simulink model "pfb_float_fft_1k_sim"  (R2016b) (continued)
== system pfb_float_fft_1k_sim == (continued)
      mask help:
      | An explicitly laid-out delay line to avoid mapping to SRL16s
      | for use in pipelining to help achieve timing closure.
      mask init:
      | pipeline_init(gcb,'latency',latency);
      settings: AttributesFormatString=Latency = %<latency>
      mask Display:
      | port_label('input', 1, 'd');
      | port_label('output', 1, 'q');
      | disp(['Z^-', num2str(latency)]);
  pipeline31 : Subsystem  AncestorBlock=casper_library_delays/pipeline  mask(pipeline){latency=2}
      mask help:
      | An explicitly laid-out delay line to avoid mapping to SRL16s
      | for use in pipelining to help achieve timing closure.
      mask init:
      | pipeline_init(gcb,'latency',latency);
      settings: AttributesFormatString=Latency = %<latency>
      mask Display:
      | port_label('input', 1, 'd');
      | port_label('output', 1, 'q');
      | disp(['Z^-', num2str(latency)]);
  pipeline34 : Subsystem  AncestorBlock=casper_library_delays/pipeline  mask(pipeline){latency=1}
      mask help:
      | An explicitly laid-out delay line to avoid mapping to SRL16s
      | for use in pipelining to help achieve timing closure.
      mask init:
      | pipeline_init(gcb,'latency',latency);
      settings: AttributesFormatString=Latency = %<latency>
      mask Display:
      | port_label('input', 1, 'd');
      | port_label('output', 1, 'q');
      | disp(['Z^-', num2str(latency)]);
  pipeline35 : Subsystem  AncestorBlock=casper_library_delays/pipeline  mask(pipeline){latency=1}
      mask help:
      | An explicitly laid-out delay line to avoid mapping to SRL16s
      | for use in pipelining to help achieve timing closure.
      mask init:
      | pipeline_init(gcb,'latency',latency);
      settings: AttributesFormatString=Latency = %<latency>
      mask Display:
      | port_label('input', 1, 'd');
      | port_label('output', 1, 'q');
      | disp(['Z^-', num2str(latency)]);
  pipeline36 : Subsystem  AncestorBlock=casper_library_delays/pipeline  mask(pipeline){latency=2}
      mask help:
      | An explicitly laid-out delay line to avoid mapping to SRL16s
      | for use in pipelining to help achieve timing closure.
      mask init:
      | pipeline_init(gcb,'latency',latency);
      settings: AttributesFormatString=Latency = %<latency>
      mask Display:
      | port_label('input', 1, 'd');
      | port_label('output', 1, 'q');
      | disp(['Z^-', num2str(latency)]);
  pipeline38 : Subsystem  AncestorBlock=casper_library_delays/pipeline  mask(pipeline){latency=2}
      mask help:
      | An explicitly laid-out delay line to avoid mapping to SRL16s
      | for use in pipelining to help achieve timing closure.
      mask init:
      | pipeline_init(gcb,'latency',latency);
      settings: AttributesFormatString=Latency = %<latency>
      mask Display:
      | port_label('input', 1, 'd');
      | port_label('output', 1, 'q');
      | disp(['Z^-', num2str(latency)]);
  pipeline39 : Subsystem  AncestorBlock=casper_library_delays/pipeline  mask(pipeline){latency=2}
      mask help:
      | An explicitly laid-out delay line to avoid mapping to SRL16s
      | for use in pipelining to help achieve timing closure.
      mask init:
      | pipeline_init(gcb,'latency',latency);
      settings: AttributesFormatString=Latency = %<latency>
      mask Display:
      | port_label('input', 1, 'd');
      | port_label('output', 1, 'q');
      | disp(['Z^-', num2str(latency)]);
  pipeline4 : Subsystem  AncestorBlock=casper_library_delays/pipeline  mask(pipeline){latency=250}
      mask help:
      | An explicitly laid-out delay line to avoid mapping to SRL16s
      | for use in pipelining to help achieve timing closure.
      mask init:
      | pipeline_init(gcb,'latency',latency);
      settings: AttributesFormatString=Latency = %<latency>
      mask Display:
      | port_label('input', 1, 'd');
      | port_label('output', 1, 'q');
      | disp(['Z^-', num2str(latency)]);
  pipeline40 : Subsystem  AncestorBlock=casper_library_delays/pipeline  mask(pipeline){latency=2}
      mask help:
      | An explicitly laid-out delay line to avoid mapping to SRL16s
      | for use in pipelining to help achieve timing closure.
      mask init:
      | pipeline_init(gcb,'latency',latency);
      settings: AttributesFormatString=Latency = %<latency>
      mask Display:
      | port_label('input', 1, 'd');
      | port_label('output', 1, 'q');
      | disp(['Z^-', num2str(latency)]);
  pipeline41 : Subsystem  AncestorBlock=casper_library_delays/pipeline  mask(pipeline){latency=2}
      mask help:
      | An explicitly laid-out delay line to avoid mapping to SRL16s
      | for use in pipelining to help achieve timing closure.
      mask init:
      | pipeline_init(gcb,'latency',latency);
      settings: AttributesFormatString=Latency = %<latency>
      mask Display:
      | port_label('input', 1, 'd');
      | port_label('output', 1, 'q');
      | disp(['Z^-', num2str(latency)]);
  pipeline42 : Subsystem  AncestorBlock=casper_library_delays/pipeline  mask(pipeline){latency=2}
      mask help:
      | An explicitly laid-out delay line to avoid mapping to SRL16s
      | for use in pipelining to help achieve timing closure.
      mask init:
      | pipeline_init(gcb,'latency',latency);
      settings: AttributesFormatString=Latency = %<latency>
      mask Display:
      | port_label('input', 1, 'd');
      | port_label('output', 1, 'q');
      | disp(['Z^-', num2str(latency)]);
  pipeline43 : Subsystem  AncestorBlock=casper_library_delays/pipeline  mask(pipeline){latency=2}
      mask help:
      | An explicitly laid-out delay line to avoid mapping to SRL16s
      | for use in pipelining to help achieve timing closure.
      mask init:
      | pipeline_init(gcb,'latency',latency);
      settings: AttributesFormatString=Latency = %<latency>
      mask Display:
      | port_label('input', 1, 'd');
      | port_label('output', 1, 'q');
      | disp(['Z^-', num2str(latency)]);
  pipeline44 : Subsystem  AncestorBlock=casper_library_delays/pipeline  mask(pipeline){latency=2}
      mask help:
      | An explicitly laid-out delay line to avoid mapping to SRL16s
      | for use in pipelining to help achieve timing closure.
      mask init:
      | pipeline_init(gcb,'latency',latency);
      settings: AttributesFormatString=Latency = %<latency>
      mask Display:
      | port_label('input', 1, 'd');
      | port_label('output', 1, 'q');
      | disp(['Z^-', num2str(latency)]);
  pipeline45 : Subsystem  AncestorBlock=casper_library_delays/pipeline  mask(pipeline){latency=2}
      mask help:
      | An explicitly laid-out delay line to avoid mapping to SRL16s
      | for use in pipelining to help achieve timing closure.
      mask init:
      | pipeline_init(gcb,'latency',latency);
      settings: AttributesFormatString=Latency = %<latency>
      mask Display:
      | port_label('input', 1, 'd');
      | port_label('output', 1, 'q');
      | disp(['Z^-', num2str(latency)]);
  pipeline46 : Subsystem  AncestorBlock=casper_library_delays/pipeline  mask(pipeline){latency=2}
      mask help:
      | An explicitly laid-out delay line to avoid mapping to SRL16s
      | for use in pipelining to help achieve timing closure.
      mask init:
      | pipeline_init(gcb,'latency',latency);
      settings: AttributesFormatString=Latency = %<latency>
      mask Display:
      | port_label('input', 1, 'd');
      | port_label('output', 1, 'q');
      | disp(['Z^-', num2str(latency)]);
  pipeline47 : Subsystem  AncestorBlock=casper_library_delays/pipeline  mask(pipeline){latency=2}
      mask help:
      | An explicitly laid-out delay line to avoid mapping to SRL16s
      | for use in pipelining to help achieve timing closure.
      mask init:
      | pipeline_init(gcb,'latency',latency);
      settings: AttributesFormatString=Latency = %<latency>
      mask Display:
      | port_label('input', 1, 'd');
      | port_label('output', 1, 'q');
      | disp(['Z^-', num2str(latency)]);
  pipeline48 : Subsystem  AncestorBlock=casper_library_delays/pipeline  mask(pipeline){latency=2}
      mask help:
      | An explicitly laid-out delay line to avoid mapping to SRL16s
      | for use in pipelining to help achieve timing closure.
      mask init:
      | pipeline_init(gcb,'latency',latency);
      settings: AttributesFormatString=Latency = %<latency>
      mask Display:
      | port_label('input', 1, 'd');
      | port_label('output', 1, 'q');
      | disp(['Z^-', num2str(latency)]);
  pipeline5 : Subsystem  AncestorBlock=casper_library_delays/pipeline  mask(pipeline){latency=2}
      mask help:
      | An explicitly laid-out delay line to avoid mapping to SRL16s
      | for use in pipelining to help achieve timing closure.
      mask init:
      | pipeline_init(gcb,'latency',latency);
      settings: AttributesFormatString=Latency = %<latency>
      mask Display:
      | port_label('input', 1, 'd');
      | port_label('output', 1, 'q');
      | disp(['Z^-', num2str(latency)]);
  pipeline53 : Subsystem  AncestorBlock=casper_library_delays/pipeline  mask(pipeline){latency=2}
      mask help:
      | An explicitly laid-out delay line to avoid mapping to SRL16s
      | for use in pipelining to help achieve timing closure.
      mask init:
      | pipeline_init(gcb,'latency',latency);
      settings: AttributesFormatString=Latency = %<latency>
      mask Display:
      | port_label('input', 1, 'd');
      | port_label('output', 1, 'q');
      | disp(['Z^-', num2str(latency)]);
  pipeline55 : Subsystem  AncestorBlock=casper_library_delays/pipeline  mask(pipeline){latency=2}
      mask help:
      | An explicitly laid-out delay line to avoid mapping to SRL16s
      | for use in pipelining to help achieve timing closure.
      mask init:
      | pipeline_init(gcb,'latency',latency);
      settings: AttributesFormatString=Latency = %<latency>
      mask Display:
      | port_label('input', 1, 'd');
      | port_label('output', 1, 'q');
      | disp(['Z^-', num2str(latency)]);
  pipeline56 : Subsystem  AncestorBlock=casper_library_delays/pipeline  mask(pipeline){latency=2}
      mask help:
      | An explicitly laid-out delay line to avoid mapping to SRL16s
      | for use in pipelining to help achieve timing closure.
      mask init:
      | pipeline_init(gcb,'latency',latency);
      settings: AttributesFormatString=Latency = %<latency>
      mask Display:
      | port_label('input', 1, 'd');
      | port_label('output', 1, 'q');
      | disp(['Z^-', num2str(latency)]);
  pipeline57 : Subsystem  AncestorBlock=casper_library_delays/pipeline  mask(pipeline){latency=1}
      mask help:
      | An explicitly laid-out delay line to avoid mapping to SRL16s
      | for use in pipelining to help achieve timing closure.
      mask init:
      | pipeline_init(gcb,'latency',latency);
      settings: AttributesFormatString=Latency = %<latency>
      mask Display:
      | port_label('input', 1, 'd');
      | port_label('output', 1, 'q');
      | disp(['Z^-', num2str(latency)]);
  pipeline58 : Subsystem  AncestorBlock=casper_library_delays/pipeline  mask(pipeline){latency=2}
      mask help:
      | An explicitly laid-out delay line to avoid mapping to SRL16s
      | for use in pipelining to help achieve timing closure.
      mask init:
      | pipeline_init(gcb,'latency',latency);
      settings: AttributesFormatString=Latency = %<latency>
      mask Display:
      | port_label('input', 1, 'd');
      | port_label('output', 1, 'q');
      | disp(['Z^-', num2str(latency)]);
  pipeline59 : Subsystem  AncestorBlock=casper_library_delays/pipeline  mask(pipeline){latency=2}
      mask help:
      | An explicitly laid-out delay line to avoid mapping to SRL16s
      | for use in pipelining to help achieve timing closure.
      mask init:
      | pipeline_init(gcb,'latency',latency);
      settings: AttributesFormatString=Latency = %<latency>
      mask Display:
      | port_label('input', 1, 'd');
      | port_label('output', 1, 'q');
      | disp(['Z^-', num2str(latency)]);
  pipeline6 : Subsystem  AncestorBlock=casper_library_delays/pipeline  mask(pipeline){latency=2}
      mask help:
      | An explicitly laid-out delay line to avoid mapping to SRL16s
      | for use in pipelining to help achieve timing closure.
      mask init:
      | pipeline_init(gcb,'latency',latency);
      settings: AttributesFormatString=Latency = %<latency>
      mask Display:
      | port_label('input', 1, 'd');
      | port_label('output', 1, 'q');
      | disp(['Z^-', num2str(latency)]);
  pipeline60 : Subsystem  AncestorBlock=casper_library_delays/pipeline  mask(pipeline){latency=2}
      mask help:
      | An explicitly laid-out delay line to avoid mapping to SRL16s
      | for use in pipelining to help achieve timing closure.
      mask init:
      | pipeline_init(gcb,'latency',latency);
      settings: AttributesFormatString=Latency = %<latency>
      mask Display:
      | port_label('input', 1, 'd');
      | port_label('output', 1, 'q');
      | disp(['Z^-', num2str(latency)]);
  pipeline61 : Subsystem  AncestorBlock=casper_library_delays/pipeline  mask(pipeline){latency=2}
      mask help:
      | An explicitly laid-out delay line to avoid mapping to SRL16s
      | for use in pipelining to help achieve timing closure.
      mask init:
      | pipeline_init(gcb,'latency',latency);
      settings: AttributesFormatString=Latency = %<latency>
      mask Display:
      | port_label('input', 1, 'd');
      | port_label('output', 1, 'q');
      | disp(['Z^-', num2str(latency)]);
  pipeline62 : Subsystem  AncestorBlock=casper_library_delays/pipeline  mask(pipeline){latency=1}
      mask help:
      | An explicitly laid-out delay line to avoid mapping to SRL16s
      | for use in pipelining to help achieve timing closure.
      mask init:
      | pipeline_init(gcb,'latency',latency);
      settings: AttributesFormatString=Latency = %<latency>
      mask Display:
      | port_label('input', 1, 'd');
      | port_label('output', 1, 'q');
      | disp(['Z^-', num2str(latency)]);
  pipeline63 : Subsystem  AncestorBlock=casper_library_delays/pipeline  mask(pipeline){latency=1}
      mask help:
      | An explicitly laid-out delay line to avoid mapping to SRL16s
      | for use in pipelining to help achieve timing closure.
      mask init:
      | pipeline_init(gcb,'latency',latency);
      settings: AttributesFormatString=Latency = %<latency>
      mask Display:
      | port_label('input', 1, 'd');
      | port_label('output', 1, 'q');
      | disp(['Z^-', num2str(latency)]);
  pipeline7 : Subsystem  AncestorBlock=casper_library_delays/pipeline  mask(pipeline){latency=2}
      mask help:
      | An explicitly laid-out delay line to avoid mapping to SRL16s
      | for use in pipelining to help achieve timing closure.
      mask init:
      | pipeline_init(gcb,'latency',latency);
      settings: AttributesFormatString=Latency = %<latency>
      mask Display:
      | port_label('input', 1, 'd');
      | port_label('output', 1, 'q');
      | disp(['Z^-', num2str(latency)]);
  pipeline79 : Subsystem  AncestorBlock=casper_library_delays/pipeline  mask(pipeline){latency=2}
      mask help:
      | An explicitly laid-out delay line to avoid mapping to SRL16s
      | for use in pipelining to help achieve timing closure.
      mask init:
      | pipeline_init(gcb,'latency',latency);
      settings: AttributesFormatString=Latency = %<latency>
      mask Display:
      | port_label('input', 1, 'd');
      | port_label('output', 1, 'q');
      | disp(['Z^-', num2str(latency)]);
  pipeline8 : Subsystem  AncestorBlock=casper_library_delays/pipeline  mask(pipeline){latency=2}
      mask help:
      | An explicitly laid-out delay line to avoid mapping to SRL16s
      | for use in pipelining to help achieve timing closure.
      mask init:
      | pipeline_init(gcb,'latency',latency);
      settings: AttributesFormatString=Latency = %<latency>
      mask Display:
      | port_label('input', 1, 'd');
      | port_label('output', 1, 'q');
      | disp(['Z^-', num2str(latency)]);
  pipeline80 : Subsystem  AncestorBlock=casper_library_delays/pipeline  mask(pipeline){latency=2}
      mask help:
      | An explicitly laid-out delay line to avoid mapping to SRL16s
      | for use in pipelining to help achieve timing closure.
      mask init:
      | pipeline_init(gcb,'latency',latency);
      settings: AttributesFormatString=Latency = %<latency>
      mask Display:
      | port_label('input', 1, 'd');
      | port_label('output', 1, 'q');
      | disp(['Z^-', num2str(latency)]);
  pipeline81 : Subsystem  AncestorBlock=casper_library_delays/pipeline  mask(pipeline){latency=2}
      mask help:
      | An explicitly laid-out delay line to avoid mapping to SRL16s
      | for use in pipelining to help achieve timing closure.
      mask init:
      | pipeline_init(gcb,'latency',latency);
      settings: AttributesFormatString=Latency = %<latency>
      mask Display:
      | port_label('input', 1, 'd');
      | port_label('output', 1, 'q');
      | disp(['Z^-', num2str(latency)]);
  pipeline82 : Subsystem  AncestorBlock=casper_library_delays/pipeline  mask(pipeline){latency=2}
      mask help:
      | An explicitly laid-out delay line to avoid mapping to SRL16s
      | for use in pipelining to help achieve timing closure.
      mask init:
      | pipeline_init(gcb,'latency',latency);
      settings: AttributesFormatString=Latency = %<latency>
      mask Display:
      | port_label('input', 1, 'd');
      | port_label('output', 1, 'q');
      | disp(['Z^-', num2str(latency)]);
  pipeline83 : Subsystem  AncestorBlock=casper_library_delays/pipeline  mask(pipeline){latency=2}
      mask help:
      | An explicitly laid-out delay line to avoid mapping to SRL16s
      | for use in pipelining to help achieve timing closure.
      mask init:
      | pipeline_init(gcb,'latency',latency);
      settings: AttributesFormatString=Latency = %<latency>
      mask Display:
      | port_label('input', 1, 'd');
      | port_label('output', 1, 'q');
      | disp(['Z^-', num2str(latency)]);
  pipeline84 : Subsystem  AncestorBlock=casper_library_delays/pipeline  mask(pipeline){latency=2}
      mask help:
      | An explicitly laid-out delay line to avoid mapping to SRL16s
      | for use in pipelining to help achieve timing closure.
      mask init:
      | pipeline_init(gcb,'latency',latency);
      settings: AttributesFormatString=Latency = %<latency>
      mask Display:
      | port_label('input', 1, 'd');
      | port_label('output', 1, 'q');
      | disp(['Z^-', num2str(latency)]);
  pipeline9 : Subsystem  AncestorBlock=casper_library_delays/pipeline  mask(pipeline){latency=2}
      mask help:
      | An explicitly laid-out delay line to avoid mapping to SRL16s
      | for use in pipelining to help achieve timing closure.
      mask init:
      | pipeline_init(gcb,'latency',latency);
      settings: AttributesFormatString=Latency = %<latency>
      mask Display:
      | port_label('input', 1, 'd');
      | port_label('output', 1, 'q');
      | disp(['Z^-', num2str(latency)]);
  pipeline96 : Subsystem  AncestorBlock=casper_library_delays/pipeline  mask(pipeline){latency=2}
      mask help:
      | An explicitly laid-out delay line to avoid mapping to SRL16s
      | for use in pipelining to help achieve timing closure.
      mask init:
      | pipeline_init(gcb,'latency',latency);
      settings: AttributesFormatString=Latency = %<latency>
      mask Display:
      | port_label('input', 1, 'd');
      | port_label('output', 1, 'q');
      | disp(['Z^-', num2str(latency)]);
  pipeline98 : Subsystem  AncestorBlock=casper_library_delays/pipeline  mask(pipeline){latency=2}
      mask help:
      | An explicitly laid-out delay line to avoid mapping to SRL16s
      | for use in pipelining to help achieve timing closure.
      mask init:
      | pipeline_init(gcb,'latency',latency);
      settings: AttributesFormatString=Latency = %<latency>
      mask Display:
      | port_label('input', 1, 'd');
      | port_label('output', 1, 'q');
      | disp(['Z^-', num2str(latency)]);
  pipeline99 : Subsystem  AncestorBlock=casper_library_delays/pipeline  mask(pipeline){latency=1}
      mask help:
      | An explicitly laid-out delay line to avoid mapping to SRL16s
      | for use in pipelining to help achieve timing closure.
      mask init:
      | pipeline_init(gcb,'latency',latency);
      settings: AttributesFormatString=Latency = %<latency>
      mask Display:
      | port_label('input', 1, 'd');
      | port_label('output', 1, 'q');
      | disp(['Z^-', num2str(latency)]);
  real_square{,1..3} (4 alike) : xbsIndex_r4/Mult  precision=Full  arith_type="Signed  (2's comp)"  n_bits=8  bin_pt=2  quantization=Truncate  overflow=Wrap  en=off  latency=6  use_behavioral_HDL=off  opt=Speed  use_embedded=on  optimum_pipeline=off  pipeline=on  dbl_ovrd=off  use_rpm=on  placement_style=Rectangular shape  block_type=mult
      settings: xl_area=[0, 0, 0, 0, 0, 0, 0]  block_version=9.1.01
  rpt_en_cwg0 : Subsystem  Tag=xps:sw_reg  AncestorBlock=xps_library/software_register  mask(swreg){io_dir=From Processor, io_delay=1, sample_period=1, names=[en dummy], bitwidths=[1 31], bin_pts=[0 0], arith_types=[0 0], sim_port=on, show_format=on}
      mask prompts: io_dir "I/O direction"; io_delay "I/O delay"; sample_period "Sample period"; names "Bitfield names [msb...lsb]"; bitwidths "Bitfield widths"; bin_pts "Bitfield binary pts"; arith_types "Bitfield types, ufix=0, fix=1, bool=2"; sim_port "Provide sim input/output?"; show_format "Print format string?"
      mask help: A 32-bit software-accessible register. Can be divided into bitfields of varying widths and types using the fields in the block mask.
      mask init:
      | swreg_init(gcb)
      settings: AttributesFormatString=2 inputs:uf1.0,uf31.0 = 32 bits
      mask TypeOptions io_dir: To Processor | From Processor
      mask Callback io_dir:
      | mask_enables = get_param(gcb, 'MaskEnables');
      | mask_names = get_param(gcb, 'MaskNames');
      | if strcmp(get_param(gcb, 'io_dir'), 'To Processor'),
      |     mask_enables{ismember(mask_names, 'sample_period')} = 'off';
      | else
      |     mask_enables{ismember(mask_names, 'sample_period')} = 'on';
      | end
      | set_param(gcb, 'MaskEnables', mask_enables);
  rpt_length_cwg0 : Subsystem  Tag=xps:sw_reg  AncestorBlock=xps_library/software_register  mask(swreg){io_dir=From Processor, io_delay=1, sample_period=1, names=cwg0_repeat_length, bitwidths=32, bin_pts=0, arith_types=0, sim_port=on, show_format=on}
      mask prompts: io_dir "I/O direction"; io_delay "I/O delay"; sample_period "Sample period"; names "Bitfield names [msb...lsb]"; bitwidths "Bitfield widths"; bin_pts "Bitfield binary pts"; arith_types "Bitfield types, ufix=0, fix=1, bool=2"; sim_port "Provide sim input/output?"; show_format "Print format string?"
      mask help: A 32-bit software-accessible register. Can be divided into bitfields of varying widths and types using the fields in the block mask.
      mask init:
      | swreg_init(gcb)
      settings: AttributesFormatString=1input:uf32.0 = 32 bits
      mask TypeOptions io_dir: To Processor | From Processor
      mask Callback io_dir:
      | mask_enables = get_param(gcb, 'MaskEnables');
      | mask_names = get_param(gcb, 'MaskNames');
      | if strcmp(get_param(gcb, 'io_dir'), 'To Processor'),
      |     mask_enables{ismember(mask_names, 'sample_period')} = 'off';
      | else
      |     mask_enables{ismember(mask_names, 'sample_period')} = 'on';
      | end
      | set_param(gcb, 'MaskEnables', mask_enables);
  scale_cwg0 : Subsystem  Tag=xps:sw_reg  AncestorBlock=xps_library/software_register  mask(swreg){io_dir=From Processor, io_delay=1, sample_period=1, names=[scale dummy], bitwidths=16, bin_pts=15, arith_types=0, sim_port=on, show_format=on}
      mask prompts: io_dir "I/O direction"; io_delay "I/O delay"; sample_period "Sample period"; names "Bitfield names [msb...lsb]"; bitwidths "Bitfield widths"; bin_pts "Bitfield binary pts"; arith_types "Bitfield types, ufix=0, fix=1, bool=2"; sim_port "Provide sim input/output?"; show_format "Print format string?"
      mask help: A 32-bit software-accessible register. Can be divided into bitfields of varying widths and types using the fields in the block mask.
      mask init:
      | swreg_init(gcb)
      settings: AttributesFormatString=2 inputs:uf16.15,uf16.15 = 32 bits
      mask TypeOptions io_dir: To Processor | From Processor
      mask Callback io_dir:
      | mask_enables = get_param(gcb, 'MaskEnables');
      | mask_names = get_param(gcb, 'MaskNames');
      | if strcmp(get_param(gcb, 'io_dir'), 'To Processor'),
      |     mask_enables{ismember(mask_names, 'sample_period')} = 'off';
      | else
      |     mask_enables{ismember(mask_names, 'sample_period')} = 'on';
      | end
      | set_param(gcb, 'MaskEnables', mask_enables);
  scale_float : Subsystem
  scale_float1 : Subsystem
  scale_float2 : Subsystem
  scale_float3 : Subsystem
  scale_wng0 : Subsystem  Tag=xps:sw_reg  AncestorBlock=xps_library/software_register  mask(swreg){io_dir=From Processor, io_delay=1, sample_period=1, names=[scale dummy], bitwidths=16, bin_pts=15, arith_types=0, sim_port=on, show_format=on}
      mask prompts: io_dir "I/O direction"; io_delay "I/O delay"; sample_period "Sample period"; names "Bitfield names [msb...lsb]"; bitwidths "Bitfield widths"; bin_pts "Bitfield binary pts"; arith_types "Bitfield types, ufix=0, fix=1, bool=2"; sim_port "Provide sim input/output?"; show_format "Print format string?"
      mask help: A 32-bit software-accessible register. Can be divided into bitfields of varying widths and types using the fields in the block mask.
      mask init:
      | swreg_init(gcb)
      settings: AttributesFormatString=2 inputs:uf16.15,uf16.15 = 32 bits
      mask TypeOptions io_dir: To Processor | From Processor
      mask Callback io_dir:
      | mask_enables = get_param(gcb, 'MaskEnables');
      | mask_names = get_param(gcb, 'MaskNames');
      | if strcmp(get_param(gcb, 'io_dir'), 'To Processor'),
      |     mask_enables{ismember(mask_names, 'sample_period')} = 'off';
      | else
      |     mask_enables{ismember(mask_names, 'sample_period')} = 'on';
      | end
      | set_param(gcb, 'MaskEnables', mask_enables);
  simple_bram_vacc : Subsystem  AncestorBlock=casper_library_accumulators/simple_bram_vacc  mask(simple_bram_vacc){vec_len=2^8, arith_type=Signed, n_bits=128, bin_pt=68}
      mask prompts: vec_len "Vector length:"; arith_type "Output type:"; n_bits "Bit width (output):"; bin_pt "Binary point (output):"
      mask help: This simple vector accumulator is based on a shift register. It outputs its previous accumulated vector after a new_acc pulse is received.
      mask init:
      | simple_bram_vacc_init(gcb, ...
      |     'vec_len', vec_len, ...
      |     'arith_type', arith_type, ...
      |     'n_bits', n_bits, ...
      |     'bin_pt', bin_pt);
      mask TypeOptions arith_type: Signed | Unsigned
  simple_bram_vacc1 : Subsystem  AncestorBlock=casper_library_accumulators/simple_bram_vacc  mask(simple_bram_vacc){vec_len=2^8, arith_type=Signed, n_bits=128, bin_pt=68}
      mask prompts: vec_len "Vector length:"; arith_type "Output type:"; n_bits "Bit width (output):"; bin_pt "Binary point (output):"
      mask help: This simple vector accumulator is based on a shift register. It outputs its previous accumulated vector after a new_acc pulse is received.
      mask init:
      | simple_bram_vacc_init(gcb, ...
      |     'vec_len', vec_len, ...
      |     'arith_type', arith_type, ...
      |     'n_bits', n_bits, ...
      |     'bin_pt', bin_pt);
      mask TypeOptions arith_type: Signed | Unsigned
  simple_bram_vacc2 : Subsystem  AncestorBlock=casper_library_accumulators/simple_bram_vacc  mask(simple_bram_vacc){vec_len=2^8, arith_type=Signed, n_bits=128, bin_pt=68}
      mask prompts: vec_len "Vector length:"; arith_type "Output type:"; n_bits "Bit width (output):"; bin_pt "Binary point (output):"
      mask help: This simple vector accumulator is based on a shift register. It outputs its previous accumulated vector after a new_acc pulse is received.
      mask init:
      | simple_bram_vacc_init(gcb, ...
      |     'vec_len', vec_len, ...
      |     'arith_type', arith_type, ...
      |     'n_bits', n_bits, ...
      |     'bin_pt', bin_pt);
      mask TypeOptions arith_type: Signed | Unsigned
  simple_bram_vacc3 : Subsystem  AncestorBlock=casper_library_accumulators/simple_bram_vacc  mask(simple_bram_vacc){vec_len=2^8, arith_type=Signed, n_bits=128, bin_pt=68}
      mask prompts: vec_len "Vector length:"; arith_type "Output type:"; n_bits "Bit width (output):"; bin_pt "Binary point (output):"
      mask help: This simple vector accumulator is based on a shift register. It outputs its previous accumulated vector after a new_acc pulse is received.
      mask init:
      | simple_bram_vacc_init(gcb, ...
      |     'vec_len', vec_len, ...
      |     'arith_type', arith_type, ...
      |     'n_bits', n_bits, ...
      |     'bin_pt', bin_pt);
      mask TypeOptions arith_type: Signed | Unsigned
  spect_cnt : xbsIndex_r4/Counter  cnt_type=Free Running  cnt_to=Inf  operation=Up  start_count=0  cnt_by_val=1  arith_type=Unsigned  n_bits=32  bin_pt=0  load_pin=off  rst=on  en=on  explicit_period=on  period=1  use_behavioral_HDL=off  implementation=DSP48  use_rpm=off  dbl_ovrd=off  block_type=counter
  spectrum_counter : Subsystem  Tag=xps:sw_reg  AncestorBlock=xps_library/software_register  mask(swreg){io_dir=To Processor, io_delay=0, sample_period=1, names=reg, bitwidths=32, bin_pts=0, arith_types=0, sim_port=on, show_format=on}
      mask prompts: io_dir "I/O direction"; io_delay "I/O delay"; sample_period "Sample period"; names "Bitfield names [msb...lsb]"; bitwidths "Bitfield widths"; bin_pts "Bitfield binary pts"; arith_types "Bitfield types, ufix=0, fix=1, bool=2"; sim_port "Provide sim input/output?"; show_format "Print format string?"
      mask help: A 32-bit software-accessible register. Can be divided into bitfields of varying widths and types using the fields in the block mask.
      mask init:
      | swreg_init(gcb)
      settings: AttributesFormatString=1output:uf32.0 = 32 bits
      mask TypeOptions io_dir: To Processor | From Processor
      mask Callback io_dir:
      | mask_enables = get_param(gcb, 'MaskEnables');
      | mask_names = get_param(gcb, 'MaskNames');
      | if strcmp(get_param(gcb, 'io_dir'), 'To Processor'),
      |     mask_enables{ismember(mask_names, 'sample_period')} = 'off';
      | else
      |     mask_enables{ismember(mask_names, 'sample_period')} = 'on';
      | end
      | set_param(gcb, 'MaskEnables', mask_enables);
  spectrum_limit : Subsystem  Tag=xps:sw_reg  AncestorBlock=xps_library/software_register  mask(swreg){io_dir=From Processor, io_delay=0, sample_period=1, names=limit, bitwidths=32, bin_pts=0, arith_types=0, sim_port=on, show_format=on}
      mask prompts: io_dir "I/O direction"; io_delay "I/O delay"; sample_period "Sample period"; names "Bitfield names [msb...lsb]"; bitwidths "Bitfield widths"; bin_pts "Bitfield binary pts"; arith_types "Bitfield types, ufix=0, fix=1, bool=2"; sim_port "Provide sim input/output?"; show_format "Print format string?"
      mask help: A 32-bit software-accessible register. Can be divided into bitfields of varying widths and types using the fields in the block mask.
      mask init:
      | swreg_init(gcb)
      settings: AttributesFormatString=1input:uf32.0 = 32 bits
      mask TypeOptions io_dir: To Processor | From Processor
      mask Callback io_dir:
      | mask_enables = get_param(gcb, 'MaskEnables');
      | mask_names = get_param(gcb, 'MaskNames');
      | if strcmp(get_param(gcb, 'io_dir'), 'To Processor'),
      |     mask_enables{ismember(mask_names, 'sample_period')} = 'off';
      | else
      |     mask_enables{ismember(mask_names, 'sample_period')} = 'on';
      | end
      | set_param(gcb, 'MaskEnables', mask_enables);
  ss_pfb_sq0 : Subsystem  Tag=casper:bitsnap  AncestorBlock=casper_library_scopes/bitfield_snapshot  mask(bitsnap){io_names=[pfb2_0], io_widths=[128], io_bps=[68], io_types=[1], show_format=on, snap_storage=bram, snap_dram_dimm=2, snap_dram_clock=250, snap_nsamples=8, snap_data_width=128, snap_offset=off, snap_circap=off, snap_value=off, snap_ext_arm=on, snap_provide_outputs=on, snap_use_dsp48=on, snap_delay=2, extra_names=[c d], extra_widths=[30 2], extra_bps=[0 0], extra_types=[0 0]}
      mask prompts: io_names "Names [msb...lsb]"; io_widths "Bitwidths [msb...lsb]"; io_bps "Binary pts [msb...lsb]"; io_types "Data types [msb...lsb], ufix=0, fix=1, bool=2"; show_format "Print format string?"; snap_storage "Storage medium"; snap_dram_dimm "DRAM dimm"; snap_dram_clock "DRAM clock rate"; snap_nsamples "Number of Samples (2^?)"; snap_data_width "Data width"; snap_offset "Start delay support"; snap_circap "Circular capture support"; snap_value "Extra value capture support"; snap_ext_arm "External arm support"; snap_provide_outputs "Provide outputs"; snap_use_dsp48 "Use DSP48s to implement counters"; snap_delay "Pre-snapshot delay"; extra_names "Names [msb...lsb]"; extra_widths "Widths [msb...lsb]"; extra_bps "Binary pts [msb...lsb]"; extra_types "Data types [msb...lsb], ufix=0, fix=1, bool=2"
      mask help:
      | A wrapped snapshot block. Allows specification of inputs to a snapblock word so the toolflow can automatically set up the scope.
      | See documentation for snapshot for explanation of parameters.
      mask init:
      | bitsnap_init(gcb);
      settings: AttributesFormatString=|
      | snap(1):f128.68 = 128 bits\n128 wide, 256 deep
      | debugID: 37190085
      mask TypeOptions snap_storage: bram | dram
      mask Callback snap_storage: bitsnap_callback();
      mask TypeOptions snap_dram_dimm: 1 | 2 | 3 | 4
      mask TypeOptions snap_dram_clock: 200 | 250 | 266 | 300 | 333
      mask TypeOptions snap_data_width: 8 | 16 | 32 | 64 | 128
      mask Callback snap_value: bitsnap_callback();
      mask Callback snap_ext_arm: bitsnap_callback();
      mask Callback snap_provide_outputs: bitsnap_callback();
  ss_pfb_sq1 : Subsystem  Tag=casper:bitsnap  AncestorBlock=casper_library_scopes/bitfield_snapshot  mask(bitsnap){io_names=[pfb2_0], io_widths=[128], io_bps=[68], io_types=[1], show_format=on, snap_storage=bram, snap_dram_dimm=2, snap_dram_clock=250, snap_nsamples=8, snap_data_width=128, snap_offset=off, snap_circap=off, snap_value=off, snap_ext_arm=off, snap_provide_outputs=on, snap_use_dsp48=on, snap_delay=2, extra_names=[c d], extra_widths=[30 2], extra_bps=[0 0], extra_types=[0 0]}
      mask prompts: io_names "Names [msb...lsb]"; io_widths "Bitwidths [msb...lsb]"; io_bps "Binary pts [msb...lsb]"; io_types "Data types [msb...lsb], ufix=0, fix=1, bool=2"; show_format "Print format string?"; snap_storage "Storage medium"; snap_dram_dimm "DRAM dimm"; snap_dram_clock "DRAM clock rate"; snap_nsamples "Number of Samples (2^?)"; snap_data_width "Data width"; snap_offset "Start delay support"; snap_circap "Circular capture support"; snap_value "Extra value capture support"; snap_ext_arm "External arm support"; snap_provide_outputs "Provide outputs"; snap_use_dsp48 "Use DSP48s to implement counters"; snap_delay "Pre-snapshot delay"; extra_names "Names [msb...lsb]"; extra_widths "Widths [msb...lsb]"; extra_bps "Binary pts [msb...lsb]"; extra_types "Data types [msb...lsb], ufix=0, fix=1, bool=2"
      mask help:
      | A wrapped snapshot block. Allows specification of inputs to a snapblock word so the toolflow can automatically set up the scope.
      | See documentation for snapshot for explanation of parameters.
      mask init:
      | bitsnap_init(gcb);
      settings: AttributesFormatString=|
      | snap(1):f128.68 = 128 bits\n128 wide, 256 deep
      | debugID: 37219876
      mask TypeOptions snap_storage: bram | dram
      mask Callback snap_storage: bitsnap_callback();
      mask TypeOptions snap_dram_dimm: 1 | 2 | 3 | 4
      mask TypeOptions snap_dram_clock: 200 | 250 | 266 | 300 | 333
      mask TypeOptions snap_data_width: 8 | 16 | 32 | 64 | 128
      mask Callback snap_value: bitsnap_callback();
      mask Callback snap_ext_arm: bitsnap_callback();
      mask Callback snap_provide_outputs: bitsnap_callback();
  ss_pfb_sq2 : Subsystem  Tag=casper:bitsnap  AncestorBlock=casper_library_scopes/bitfield_snapshot  mask(bitsnap){io_names=[pfb2_0], io_widths=[128], io_bps=[68], io_types=[1], show_format=on, snap_storage=bram, snap_dram_dimm=2, snap_dram_clock=250, snap_nsamples=8, snap_data_width=128, snap_offset=off, snap_circap=off, snap_value=off, snap_ext_arm=off, snap_provide_outputs=on, snap_use_dsp48=on, snap_delay=2, extra_names=[c d], extra_widths=[30 2], extra_bps=[0 0], extra_types=[0 0]}
      mask prompts: io_names "Names [msb...lsb]"; io_widths "Bitwidths [msb...lsb]"; io_bps "Binary pts [msb...lsb]"; io_types "Data types [msb...lsb], ufix=0, fix=1, bool=2"; show_format "Print format string?"; snap_storage "Storage medium"; snap_dram_dimm "DRAM dimm"; snap_dram_clock "DRAM clock rate"; snap_nsamples "Number of Samples (2^?)"; snap_data_width "Data width"; snap_offset "Start delay support"; snap_circap "Circular capture support"; snap_value "Extra value capture support"; snap_ext_arm "External arm support"; snap_provide_outputs "Provide outputs"; snap_use_dsp48 "Use DSP48s to implement counters"; snap_delay "Pre-snapshot delay"; extra_names "Names [msb...lsb]"; extra_widths "Widths [msb...lsb]"; extra_bps "Binary pts [msb...lsb]"; extra_types "Data types [msb...lsb], ufix=0, fix=1, bool=2"
      mask help:
      | A wrapped snapshot block. Allows specification of inputs to a snapblock word so the toolflow can automatically set up the scope.
      | See documentation for snapshot for explanation of parameters.
      mask init:
      | bitsnap_init(gcb);
      settings: AttributesFormatString=|
      | snap(1):f128.68 = 128 bits\n128 wide, 256 deep
      | debugID: 37249667
      mask TypeOptions snap_storage: bram | dram
      mask Callback snap_storage: bitsnap_callback();
      mask TypeOptions snap_dram_dimm: 1 | 2 | 3 | 4
      mask TypeOptions snap_dram_clock: 200 | 250 | 266 | 300 | 333
      mask TypeOptions snap_data_width: 8 | 16 | 32 | 64 | 128
      mask Callback snap_value: bitsnap_callback();
      mask Callback snap_ext_arm: bitsnap_callback();
      mask Callback snap_provide_outputs: bitsnap_callback();
  ss_pfb_sq3 : Subsystem  Tag=casper:bitsnap  AncestorBlock=casper_library_scopes/bitfield_snapshot  mask(bitsnap){io_names=[pfb2_0], io_widths=[128], io_bps=[68], io_types=[1], show_format=on, snap_storage=bram, snap_dram_dimm=2, snap_dram_clock=250, snap_nsamples=8, snap_data_width=128, snap_offset=off, snap_circap=off, snap_value=off, snap_ext_arm=off, snap_provide_outputs=on, snap_use_dsp48=on, snap_delay=2, extra_names=[c d], extra_widths=[30 2], extra_bps=[0 0], extra_types=[0 0]}
      mask prompts: io_names "Names [msb...lsb]"; io_widths "Bitwidths [msb...lsb]"; io_bps "Binary pts [msb...lsb]"; io_types "Data types [msb...lsb], ufix=0, fix=1, bool=2"; show_format "Print format string?"; snap_storage "Storage medium"; snap_dram_dimm "DRAM dimm"; snap_dram_clock "DRAM clock rate"; snap_nsamples "Number of Samples (2^?)"; snap_data_width "Data width"; snap_offset "Start delay support"; snap_circap "Circular capture support"; snap_value "Extra value capture support"; snap_ext_arm "External arm support"; snap_provide_outputs "Provide outputs"; snap_use_dsp48 "Use DSP48s to implement counters"; snap_delay "Pre-snapshot delay"; extra_names "Names [msb...lsb]"; extra_widths "Widths [msb...lsb]"; extra_bps "Binary pts [msb...lsb]"; extra_types "Data types [msb...lsb], ufix=0, fix=1, bool=2"
      mask help:
      | A wrapped snapshot block. Allows specification of inputs to a snapblock word so the toolflow can automatically set up the scope.
      | See documentation for snapshot for explanation of parameters.
      mask init:
      | bitsnap_init(gcb);
      settings: AttributesFormatString=|
      | snap(1):f128.68 = 128 bits\n128 wide, 256 deep
      | debugID: 37279458
      mask TypeOptions snap_storage: bram | dram
      mask Callback snap_storage: bitsnap_callback();
      mask TypeOptions snap_dram_dimm: 1 | 2 | 3 | 4
      mask TypeOptions snap_dram_clock: 200 | 250 | 266 | 300 | 333
      mask TypeOptions snap_data_width: 8 | 16 | 32 | 64 | 128
      mask Callback snap_value: bitsnap_callback();
      mask Callback snap_ext_arm: bitsnap_callback();
      mask Callback snap_provide_outputs: bitsnap_callback();
  sys_en : Subsystem  Tag=xps:sw_reg  AncestorBlock=xps_library/software_register  mask(swreg){io_dir=From Processor, io_delay=0, sample_period=1, names=en, bitwidths=1, bin_pts=0, arith_types=2, sim_port=on, show_format=on}
      mask prompts: io_dir "I/O direction"; io_delay "I/O delay"; sample_period "Sample period"; names "Bitfield names [msb...lsb]"; bitwidths "Bitfield widths"; bin_pts "Bitfield binary pts"; arith_types "Bitfield types, ufix=0, fix=1, bool=2"; sim_port "Provide sim input/output?"; show_format "Print format string?"
      mask help: A 32-bit software-accessible register. Can be divided into bitfields of varying widths and types using the fields in the block mask.
      mask init:
      | swreg_init(gcb)
      settings: AttributesFormatString=1input:b = 1 bits
      mask TypeOptions io_dir: To Processor | From Processor
      mask Callback io_dir:
      | mask_enables = get_param(gcb, 'MaskEnables');
      | mask_names = get_param(gcb, 'MaskNames');
      | if strcmp(get_param(gcb, 'io_dir'), 'To Processor'),
      |     mask_enables{ismember(mask_names, 'sample_period')} = 'off';
      | else
      |     mask_enables{ismember(mask_names, 'sample_period')} = 'on';
      | end
      | set_param(gcb, 'MaskEnables', mask_enables);
  sys_rst : Subsystem  Tag=xps:sw_reg  AncestorBlock=xps_library/software_register  mask(swreg){io_dir=From Processor, io_delay=0, sample_period=1, names=rst, bitwidths=1, bin_pts=0, arith_types=2, sim_port=on, show_format=on}
      mask prompts: io_dir "I/O direction"; io_delay "I/O delay"; sample_period "Sample period"; names "Bitfield names [msb...lsb]"; bitwidths "Bitfield widths"; bin_pts "Bitfield binary pts"; arith_types "Bitfield types, ufix=0, fix=1, bool=2"; sim_port "Provide sim input/output?"; show_format "Print format string?"
      mask help: A 32-bit software-accessible register. Can be divided into bitfields of varying widths and types using the fields in the block mask.
      mask init:
      | swreg_init(gcb)
      settings: AttributesFormatString=1input:b = 1 bits
      mask TypeOptions io_dir: To Processor | From Processor
      mask Callback io_dir:
      | mask_enables = get_param(gcb, 'MaskEnables');
      | mask_names = get_param(gcb, 'MaskNames');
      | if strcmp(get_param(gcb, 'io_dir'), 'To Processor'),
      |     mask_enables{ismember(mask_names, 'sample_period')} = 'off';
      | else
      |     mask_enables{ismember(mask_names, 'sample_period')} = 'on';
      | end
      | set_param(gcb, 'MaskEnables', mask_enables);
  tvg_sel1 : Subsystem  Tag=xps:sw_reg  AncestorBlock=xps_library/software register  mask(swreg){io_dir=From Processor, io_delay=0, sample_period=1, names=[tvg_sel], bitwidths=[1], bin_pts=0, arith_types=[0], sim_port=on, show_format=on}
      mask prompts: io_dir "I/O direction"; io_delay "I/O delay"; sample_period "Sample period"; names "Bitfield names [msb...lsb]"; bitwidths "Bitfield widths"; bin_pts "Bitfield binary pts"; arith_types "Bitfield types, ufix=0, fix=1, bool=2"; sim_port "Provide sim input/output?"; show_format "Print format string?"
      mask help: A 32-bit software-accessible register. Can be divided into bitfields of varying widths and types using the fields in the block mask.
      mask init:
      | swreg_init(gcb)
      settings: AttributesFormatString=1input:uf1.0 = 1 bits
      mask TypeOptions io_dir: To Processor | From Processor
      mask Callback io_dir:
      | mask_enables = get_param(gcb, 'MaskEnables');
      | mask_names = get_param(gcb, 'MaskNames');
      | if strcmp(get_param(gcb, 'io_dir'), 'To Processor'),
      |     mask_enables{ismember(mask_names, 'sample_period')} = 'off';
      | else
      |     mask_enables{ismember(mask_names, 'sample_period')} = 'on';
      | end
      | set_param(gcb, 'MaskEnables', mask_enables);
  wng0 : Subsystem
  tag scope: acc_len=global, acc_scale=global, cnt_rst=global
  wires:
  pfb/2(dout0) -> pipeline81/1(d)
  pfb/3(dout1) -> pipeline82/1(d)
  [fft_shift] -> pfb/1(shift)
  pfb/5(of) -> pipeline84/1(d)
  pfb/4(dvalid) -> pipeline83/1(d)
  pfb/1(sync_out) -> pipeline80/1(d)
  [pfbin_d1] -> pfb/4(din1)
  [pfbin_sync] -> pfb/2(sync_in)
  [pfbin_dv] -> pfb/5(en)
  fft_shift/1(in_fft_shift) -> [fft_shift]
  Gateway Out8/1 -> To Workspace1/1
  [pfb_sync] -> Gateway Out8/1
  Gateway Out9/1 -> To Workspace10/1
  [pfb_dv] -> Gateway Out9/1
  Constant3/1 -> fft_shift/1(sim_1)
  [pfbin_d0] -> pfb/3(din0)
  Gateway Out51/1 -> To Workspace3/1
  [pfb_of] -> Gateway Out51/1
  Counter3/1 -> Relational1/1
  Constant/1 -> Relational1/2
  [local_rst] -> edge_detect5/1
  edge_detect5/1 -> Counter7/1
  spectrum_counter/1(sim_out) -> Terminator16/1
  Constant48/1 -> spectrum_limit/1(sim_1)
  spectrum_limit/1(in_limit) -> [spec_limit]
  Counter7/1 -> [spec_counter], Gateway Out88/1
  [spec_counter] -> pipeline6/1(d)
  Gateway Out88/1 -> To Workspace88/1
  Relational1/1 -> edge_detect2/1
  [local_rst] -> edge_detect3/1
  [spec_trig] -> Counter7/2
  Constant4/1 -> sys_rst/1(sim_1)
  sys_rst/1(in_rst) -> pipeline8/1(d)
  pipeline8/1(q) -> edge_detect1/1
  edge_detect1/1 -> [rst]
  [rst] -> Counter4/1
  Counter4/1 -> pipeline9/1(d)
  pfbin_sync_count/1(sim_out) -> Terminator17/1
  pipeline9/1(q) -> pfbin_sync_count/1(out_reg), Gateway Out69/1
  Gateway Out69/1 -> To Workspace69/1
  [pfbin_sync] -> Counter4/2
  [rst] -> Counter5/1
  Counter5/1 -> pipeline10/1(d)
  pfbout_sync_count/1(sim_out) -> Terminator18/1
  pipeline10/1(q) -> Gateway Out70/1, pfbout_sync_count/1(out_reg)
  Gateway Out70/1 -> To Workspace70/1
  [pfb_sync] -> Counter5/2
  edge_detect3/1 -> Counter3/1
  edge_detect2/1 -> [spec_trig]
  [pfb_dv] -> Counter3/2
  pfb_of/1(sim_out) -> Terminator9/1
  pipeline6/1(q) -> spectrum_counter/1(out_reg)
  pipeline7/1(q) -> pfb_of/1(out_reg)
  Constant29/1 -> sys_en/1(sim_1)
  sys_en/1(in_en) -> pipeline4/1(d)
  [pfb_of] -> pipeline7/1(d)
  [pfb_sync] -> Logical12/1
  [rst] -> Logical12/2
  Logical12/1 -> [local_rst]
  pipeline4/1(q) -> pipeline12/1(d)
  pipeline12/1(q) -> [sys_en]
  pipeline81/1(q) -> [pfb_dfloat_0]
  pipeline82/1(q) -> [pfb_dfloat_1]
  pipeline84/1(q) -> [pfb_of]
  pipeline80/1(q) -> [pfb_sync]
  pipeline83/1(q) -> [pfb_dv]
  Gateway Out44/1 -> To Workspace72/1
  Gateway Out47/1 -> To Workspace73/1
  Gateway Out52/1 -> To Workspace74/1
  Gateway Out43/1 -> To Workspace92/1
  Gateway Out45/1 -> To Workspace93/1
  Gateway Out50/1 -> To Workspace94/1
  Gateway Out53/1 -> To Workspace95/1
  Gateway Out40/1 -> To Workspace71/1
  bus_expand1/1(msb_out8) -> Reinterpret/1
  bus_expand1/2(out7) -> Reinterpret1/1
  bus_expand1/3(out6) -> Reinterpret2/1
  bus_expand1/4(out5) -> Reinterpret3/1
  bus_expand1/5(out4) -> Reinterpret4/1
  bus_expand1/6(out3) -> Reinterpret5/1
  bus_expand1/7(out2) -> Reinterpret6/1
  bus_expand1/8(lsb_out1) -> Reinterpret7/1
  [pfb_dfloat_0] -> bus_expand1/1(bus_in)
  Reinterpret/1 -> Gateway Out40/1
  Reinterpret1/1 -> Gateway Out43/1
  Reinterpret2/1 -> Gateway Out44/1
  Reinterpret3/1 -> Gateway Out45/1
  Reinterpret5/1 -> Gateway Out50/1
  Reinterpret4/1 -> Gateway Out47/1
  Reinterpret6/1 -> Gateway Out52/1
  Reinterpret7/1 -> Gateway Out53/1
  acc_cntrl/1(new_acc) -> Gateway Out25/1, [new_acc]
  [acc_len] -> acc_cntrl/1(acc_len)
  pipeline53/1(q) -> Delay7/1
  Delay34/1 -> Delay26/1
  Delay24/1 -> acc_cntrl/2(sync)
  cnt_rst/1(in_reg) -> pipeline19/1(d)
  Constant14/1 -> cnt_rst/1(sim_1)
  Constant16/1 -> acc_len/1(sim_1)
  acc_len/1(in_reg) -> pipeline13/1(d)
  pipeline19/1(q) -> Slice2/1
  Slice2/1 -> [cnt_rst]
  pipeline13/1(q) -> [acc_len]
  Logical/1 -> acc_cntrl/3(rst)
  [cnt_rst] -> Logical/2
  [pfb_sync] -> pipeline53/1(d)
  [pfb_r0] -> Reinterpret8/1
  [pfb_i0] -> Reinterpret9/1
  [pfb_r1] -> Reinterpret10/1
  [pfb_i1] -> Reinterpret11/1
  [pfb_r2] -> Reinterpret12/1
  [pfb_i2] -> Reinterpret13/1
  [pfb_r3] -> Reinterpret14/1
  [pfb_i3] -> Reinterpret15/1
  Delay26/1 -> Delay27/1
  Constant13/1 -> acc_scale/1(sim_1)
  acc_scale/1(in_reg) -> pipeline58/1(d)
  pipeline58/1(q) -> [acc_scale]
  simple_bram_vacc/2(valid) -> [acc_dv], edge_detect8/1
  simple_bram_vacc/1(dout) -> [acc_d0]
  pipeline24/1(q) -> pipeline20/1(d)
  pipeline18/1(q) -> simple_bram_vacc/1(new_acc)
  pipeline20/1(q) -> simple_bram_vacc/2(din)
  pipeline17/1(q) -> spect_cnt/1
  [new_acc] -> pipeline18/1(d)
  Gateway Out15/1 -> To Workspace16/1
  Gateway Out16/1 -> To Workspace17/1
  Gateway Out17/1 -> To Workspace18/1
  pipeline23/1(q) -> pipeline22/1(d)
  pipeline21/1(q) -> simple_bram_vacc1/1(new_acc)
  pipeline22/1(q) -> simple_bram_vacc1/2(din)
  [new_acc] -> pipeline21/1(d)
  Gateway Out27/1 -> To Workspace26/1
  pipeline31/1(q) -> pipeline30/1(d)
  pipeline14/1(q) -> simple_bram_vacc2/1(new_acc)
  pipeline30/1(q) -> simple_bram_vacc2/2(din)
  [new_acc] -> pipeline14/1(d)
  Gateway Out1/1 -> To Workspace4/1
  pipeline36/1(q) -> pipeline35/1(d)
  pipeline34/1(q) -> simple_bram_vacc3/1(new_acc)
  pipeline35/1(q) -> simple_bram_vacc3/2(din)
  [new_acc] -> pipeline34/1(d)
  Gateway Out57/1 -> To Workspace52/1
  spect_cnt/1 -> [acc]
  [acc] -> Gateway Out15/1
  [acc_dv] -> Gateway Out17/1
  simple_bram_vacc1/1(dout) -> [acc_d1]
  simple_bram_vacc2/1(dout) -> [acc_d2]
  simple_bram_vacc3/1(dout) -> [acc_d3]
  ss_pfb_sq0/1(arm_out) -> Terminator4/1
  edge_detect9/1 -> ss_pfb_sq0/3(trig)
  [acc_d1] -> pipeline59/1(d)
  edge_detect4/1 -> ss_pfb_sq1/3(trig)
  [acc_d2] -> pipeline16/1(d)
  edge_detect6/1 -> ss_pfb_sq2/3(trig)
  [acc_d3] -> pipeline96/1(d)
  edge_detect7/1 -> ss_pfb_sq3/3(trig)
  simple_bram_vacc1/2(valid) -> Terminator8/1
  simple_bram_vacc3/2(valid) -> Terminator12/1
  Delay25/1 -> pipeline24/1(d)
  Delay9/1 -> pipeline23/1(d)
  Delay8/1 -> pipeline31/1(d)
  Delay16/1 -> pipeline36/1(d)
  real_square/1 -> AddSub/1
  real_square1/1 -> AddSub1/1
  imag_square1/1 -> AddSub1/2
  real_square2/1 -> AddSub2/1
  imag_square2/1 -> AddSub2/2
  real_square3/1 -> AddSub3/1
  imag_square3/1 -> AddSub3/2
  AddSub1/1 -> Gateway Out10/1, Convert1/1
  AddSub2/1 -> Gateway Out12/1, Convert2/1
  AddSub3/1 -> Gateway Out21/1, Convert3/1
  [acc_d0] -> pipeline55/1(d)
  [acc_d0] -> Gateway Out16/1
  [acc_d1] -> Gateway Out27/1
  [acc_d2] -> Gateway Out1/1
  [acc_d3] -> Gateway Out57/1
  pipeline56/1(q) -> ss_pfb_sq0/2(we)
  pipeline57/1(q) -> edge_detect9/1
  pipeline61/1(q) -> ss_pfb_sq1/2(we)
  pipeline62/1(q) -> edge_detect4/1
  pipeline60/1(q) -> ss_pfb_sq2/2(we)
  pipeline63/1(q) -> edge_detect6/1
  pipeline99/1(q) -> edge_detect7/1
  pipeline98/1(q) -> ss_pfb_sq3/2(we)
  pipeline59/1(q) -> ss_pfb_sq1/1(in_pfb2_0)
  pipeline16/1(q) -> ss_pfb_sq2/1(in_pfb2_0)
  pipeline96/1(q) -> ss_pfb_sq3/1(in_pfb2_0)
  scale_float/1(real_out) -> real_square/2, real_square/1
  scale_float/2(imag_out) -> imag_square/1, imag_square/2
  Convert4/1 -> Gateway Out7/1, Delay25/1
  pipeline55/1(q) -> ss_pfb_sq0/1(in_pfb2_0)
  Convert1/1 -> Gateway Out11/1, Delay9/1
  Convert2/1 -> Gateway Out18/1, Delay8/1
  Convert3/1 -> Gateway Out22/1, Delay16/1
  scale_float2/1(real_out) -> real_square2/2, real_square2/1
  scale_float2/2(imag_out) -> imag_square2/1, imag_square2/2
  scale_float3/1(real_out) -> real_square3/2, real_square3/1
  scale_float3/2(imag_out) -> imag_square3/1, imag_square3/2
  scale_float1/1(real_out) -> real_square1/2, real_square1/1
  scale_float1/2(imag_out) -> imag_square1/1, imag_square1/2
  Reinterpret8/1 -> scale_float/1(real)
  Reinterpret9/1 -> scale_float/2(imag)
  [acc_scale] -> scale_float/3(scale)
  Reinterpret10/1 -> scale_float1/1(real)
  Reinterpret11/1 -> scale_float1/2(imag)
  [acc_scale] -> scale_float1/3(scale)
  Reinterpret12/1 -> scale_float2/1(real)
  Reinterpret13/1 -> scale_float2/2(imag)
  [acc_scale] -> scale_float2/3(scale)
  Reinterpret14/1 -> scale_float3/1(real)
  Reinterpret15/1 -> scale_float3/2(imag)
  [acc_scale] -> scale_float3/3(scale)
  Delay7/1 -> Delay34/1
  Delay27/1 -> Logical/1, Delay24/1
  Gateway Out2/1 -> To Workspace5/1
  bus_expand2/1(msb_out8) -> Gateway Out2/1
  Gateway Out3/1 -> To Workspace6/1
  bus_expand2/2(out7) -> Gateway Out3/1
  Gateway Out4/1 -> To Workspace7/1
  Gateway Out5/1 -> To Workspace14/1
  bus_expand2/4(out5) -> Gateway Out5/1
  bus_expand2/3(out6) -> Gateway Out4/1
  Gateway Out13/1 -> To Workspace19/1
  Gateway Out14/1 -> To Workspace15/1
  bus_expand2/6(out3) -> Gateway Out14/1
  bus_expand2/5(out4) -> Gateway Out13/1
  Gateway Out19/1 -> To Workspace21/1
  Gateway Out20/1 -> To Workspace20/1
  bus_expand2/7(out2) -> Gateway Out19/1
  bus_expand2/8(lsb_out1) -> Gateway Out20/1
  [pfb_dfloat_0] -> float_to_fix/1(In1)
  [acc_scale] -> float_to_fix/2(In2)
  float_to_fix/1(Out1) -> bus_expand2/1(bus_in)
  bus_expand10/2(out7) -> pipeline42/1(d)
  bus_expand10/4(out5) -> pipeline39/1(d)
  bus_expand10/6(out3) -> pipeline15/1(d)
  bus_expand10/8(lsb_out1) -> pipeline46/1(d)
  bus_expand10/7(out2) -> pipeline11/1(d)
  bus_expand10/5(out4) -> pipeline38/1(d)
  bus_expand10/3(out6) -> pipeline40/1(d)
  bus_expand10/1(msb_out8) -> pipeline43/1(d)
  [pfb_dfloat_0] -> bus_expand10/1(bus_in)
  pipeline43/1(q) -> [pfb_r0]
  pipeline42/1(q) -> [pfb_i0]
  pipeline40/1(q) -> [pfb_r1]
  pipeline39/1(q) -> [pfb_i1]
  pipeline38/1(q) -> [pfb_r2]
  pipeline15/1(q) -> [pfb_i2]
  pipeline11/1(q) -> [pfb_r3]
  pipeline46/1(q) -> [pfb_i3]
  imag_square/1 -> AddSub/2
  AddSub/1 -> Gateway Out6/1, Convert4/1
  Constant35/1 -> scale_wng0/1(sim_1)
  pipeline41/1(q) -> wng0/1(scale_16_14)
  wng0/1(bus_out) -> bus_addsub4/1(a), bus_expand4/1(bus_in)
  cwg0/1(bus_out) -> bus_addsub4/2(b)
  freq_cwg0/1(in_frequency) -> pipeline45/1(d)
  Constant37/1 -> scale_cwg0/2(sim_2)
  Constant72/1 -> scale_cwg0/1(sim_1)
  pipeline45/1(q) -> cwg0/1(freq)
  pipeline44/1(q) -> cwg0/2(scale)
  [mrst] -> pipeline180/1(d)
  Constant60/1 -> rpt_en_cwg0/2(sim_2)
  Constant62/1 -> rpt_length_cwg0/1(sim_1)
  rpt_en_cwg0/1(in_en) -> Convert25/1
  rpt_en_cwg0/2(in_dummy) -> Terminator57/1
  Constant70/1 -> rpt_en_cwg0/1(sim_1)
  Convert25/1 -> pipeline181/1(d)
  rpt_length_cwg0/1(in_cwg0_repeat_length) -> pipeline182/1(d)
  pipeline180/1(q) -> cwg0/3(rst)
  pipeline181/1(q) -> cwg0/4(repeat_en)
  pipeline182/1(q) -> cwg0/5(repeat_length)
  cwg0/3(rpt_rst) -> Terminator61/1
  pipeline198/1(q) -> wng0/2(rst)
  Constant23/1 -> man_rst_wng0/1(sim_1)
  Logical20/1 -> pipeline198/1(d)
  man_rst_wng0/1(in_rst) -> Logical20/2
  [wng0_rst] -> Logical20/1
  Gateway Out24/1 -> To Workspace24/1
  Constant103/1 -> scale_wng0/2(sim_2)
  Constant106/1 -> cwg0/6(en)
  cwg0/2(dvalid) -> Terminator28/1
  scale_wng0/1(in_scale) -> Gateway Out24/1, pipeline41/1(d)
  scale_cwg0/1(in_scale) -> Gateway Out23/1, pipeline44/1(d)
  Gateway Out23/1 -> To Workspace29/1
  Constant6/1 -> [wng0_rst]
  Constant17/1 -> [mrst]
  Gateway Out29/1 -> To Workspace30/1
  bus_expand5/1(msb_out8) -> Gateway Out29/1
  Gateway Out37/1 -> To Workspace32/1
  bus_expand5/8(lsb_out1) -> Gateway Out37/1
  Gateway Out36/1 -> To Workspace33/1
  bus_expand5/7(out2) -> Gateway Out36/1
  Gateway Out35/1 -> To Workspace34/1
  bus_expand5/6(out3) -> Gateway Out35/1
  Gateway Out34/1 -> To Workspace35/1
  bus_expand5/5(out4) -> Gateway Out34/1
  Gateway Out33/1 -> To Workspace36/1
  bus_expand5/4(out5) -> Gateway Out33/1
  Gateway Out32/1 -> To Workspace37/1
  bus_expand5/3(out6) -> Gateway Out32/1
  Gateway Out31/1 -> To Workspace38/1
  bus_expand5/2(out7) -> Gateway Out31/1
  Constant11/1 -> Terminator1/1
  Constant5/1 -> tvg_sel1/1(sim_1)
  tvg_sel1/1(in_tvg_sel) -> [tvg_sel]
  Gateway Out55/1 -> To Workspace40/1
  bus_expand4/1(msb_out8) -> Gateway Out55/1
  bus_expand4/8(lsb_out1) -> Gateway Out63/1
  bus_expand4/7(out2) -> Gateway Out62/1
  bus_expand4/6(out3) -> Gateway Out61/1
  bus_expand4/5(out4) -> Gateway Out60/1
  bus_expand4/4(out5) -> Gateway Out59/1
  bus_expand4/3(out6) -> Gateway Out58/1
  bus_expand4/2(out7) -> Gateway Out56/1
  Gateway Out56/1 -> To Workspace56/1
  Gateway Out58/1 -> To Workspace55/1
  Gateway Out59/1 -> To Workspace54/1
  Gateway Out60/1 -> To Workspace53/1
  Gateway Out61/1 -> To Workspace51/1
  Gateway Out62/1 -> To Workspace50/1
  Gateway Out63/1 -> To Workspace49/1
  bus_addsub4/1(dout) -> pipeline48/1(d)
  bus_convert/1(dout) -> bus_expand5/1(bus_in), pipeline47/1(d)
  pipeline47/1(q) -> [cwg_80_0]
  pipeline48/1(q) -> bus_convert/1(din)
  edge_detect/1 -> pipeline1/1(d)
  pipeline1/1(q) -> [pfbin_sync]
  pipeline2/1(q) -> [pfbin_d0]
  pipeline3/1(q) -> [pfbin_d1]
  pipeline5/1(q) -> [pfbin_dv]
  [cwg_80_0] -> pipeline3/1(d), pipeline2/1(d)
  Counter/1 -> Relational/1
  Constant7/1 -> Relational/2
  Relational/1 -> Register/1, Register/3
  Register/1 -> pipeline79/1(d)
  [rst] -> Register/2, Counter/1
  [sys_en] -> Counter/2
  pipeline79/1(q) -> pipeline5/1(d), edge_detect/1
  Constant20/1 -> forty_gbe/9(debug_rst)
  Constant19/1 -> forty_gbe/8(rx_overrun_ack)
  Constant46/1 -> forty_gbe/3(tx_valid)
  Constant47/1 -> forty_gbe/2(tx_data)
  Constant21/1 -> forty_gbe/6(tx_end_of_frame)
  Constant49/1 -> forty_gbe/4(tx_dest_ip)
  Constant18/1 -> forty_gbe/7(rx_ack)
  Constant50/1 -> forty_gbe/5(tx_dest_port)
  [rst] -> forty_gbe/1(rst)
  simple_bram_vacc2/2(valid) -> Terminator11/1
  ss_pfb_sq3/1(arm_out) -> Terminator7/1
  ss_pfb_sq2/1(arm_out) -> Terminator6/1
  ss_pfb_sq1/1(arm_out) -> Terminator5/1
  Constant10/1 -> freq_cwg0/1(sim_1)
  Gateway Out6/1 -> To Workspace2/1
  Gateway Out7/1 -> To Workspace8/1
  Gateway Out10/1 -> To Workspace9/1
  Gateway Out11/1 -> To Workspace11/1
  Gateway Out12/1 -> To Workspace12/1
  Gateway Out18/1 -> To Workspace13/1
  Gateway Out21/1 -> To Workspace22/1
  Gateway Out22/1 -> To Workspace23/1
  Gateway Out25/1 -> To Workspace25/1
  edge_detect8/1 -> spect_cnt/2
  [pfb_sync] -> pipeline17/1(d)
  [acc] -> Relational2/1
  Constant1/1 -> Relational2/2
  Relational2/1 -> [acc_ss_valid]
  Logical1/1 -> pipeline56/1(d), pipeline57/1(d)
  [acc_dv] -> Logical1/1
  [acc_ss_valid] -> Logical1/2
  [acc_dv] -> Logical2/1
  [acc_ss_valid] -> Logical2/2
  Logical2/1 -> pipeline61/1(d), pipeline62/1(d)
  [acc_dv] -> Logical3/1
  [acc_ss_valid] -> Logical3/2
  Logical3/1 -> pipeline60/1(d), pipeline63/1(d)
  [acc_dv] -> Logical4/1
  [acc_ss_valid] -> Logical4/2
  Logical4/1 -> pipeline98/1(d), pipeline99/1(d)
  Gateway Out26/1 -> To Workspace27/1
  [acc_ss_valid] -> Gateway Out26/1
